simulink library "inp"  (Simulink 8.2)
settings: Solver=ode45  SolverMode=SingleTasking  StartTime=0.0  StopTime=10.0  RelTol=1e-3
undefined symbols (not defined in the file or in its folder): C_TICK_TIME_S, ENUM_FAULTSTATE_FAIL, ENUM_FAULTSTATE_INDETERMINATE, ENUM_FAULTSTATE_PASS, ENUM_KEYSWITCH_ON, FALSE, KINP_ABS1CANCommuOk_flg_ovrdflg, KINP_ABS1CANCommuOk_flg_ovrdval, KINP_ABS1CANPeriodTime_s, KINP_ABS1CANRolCntOk_flg_ovrdflg, KINP_ABS1CANRolCntOk_flg_ovrdval, KINP_ABS2CANChkSumOk_flg_ovrdflg, KINP_ABS2CANChkSumOk_flg_ovrdval, KINP_ABS2CANCommuOk_flg_ovrdflg, KINP_ABS2CANCommuOk_flg_ovrdval, KINP_ABS2CANPeriodTime_s, KINP_ABS2CANRolCntOk_flg_ovrdflg, KINP_ABS2CANRolCntOk_flg_ovrdval, KINP_ABS3CANCommuOk_flg_ovrdflg, KINP_ABS3CANCommuOk_flg_ovrdval, KINP_ABS4CANCommuOk_flg_ovrdflg, KINP_ABS4CANCommuOk_flg_ovrdval, KINP_ABSActive_flg_defval, KINP_ABSActive_flg_invalid, KINP_ABSActive_flg_ovrdflg, KINP_ABSActive_flg_ovrdval, KINP_ABSActive_flg_setvalid, KINP_ABSActive_flg_srvcflg, KINP_ABSActive_flg_srvcval, KINP_ABSCANAlive_flg_ovrdflg, KINP_ABSCANAlive_flg_ovrdval, KINP_ABSCANCommuOk_flg_ovrdflg, KINP_ABSCANCommuOk_flg_ovrdval, KINP_ABSCANValidOk_flg_ovrdflg, KINP_ABSCANValidOk_flg_ovrdval, KINP_ABSFault_flg_defval, KINP_ABSFault_flg_invalid, KINP_ABSFault_flg_ovrdflg, KINP_ABSFault_flg_ovrdval, KINP_ABSFault_flg_setvalid, KINP_ABSFault_flg_srvcflg, KINP_ABSFault_flg_srvcval, KINP_AC1CANCommuOk_flg_ovrdflg, KINP_AC1CANCommuOk_flg_ovrdval, KINP_ACCANAlive_flg_ovrdflg, KINP_ACCANAlive_flg_ovrdval, KINP_ACCANCommuOk_flg_ovrdflg, KINP_ACCANCommuOk_flg_ovrdval, KINP_ACCANValidOk_flg_ovrdflg, KINP_ACCANValidOk_flg_ovrdval, KINP_ACCCancelH_flg_defval, KINP_ACCCancelH_flg_invalid, KINP_ACCCancelH_flg_ovrdflg, KINP_ACCCancelH_flg_ovrdval, KINP_ACCCancelH_flg_setvalid, KINP_ACCCancelH_flg_srvcflg, KINP_ACCCancelH_flg_srvcval, KINP_AccelPedalPosn_pct_defval, KINP_AccelPedalPosn_pct_gain, KINP_AccelPedalPosn_pct_invalid ... (+1866)
libraries: inp x192, hcu_lib x34, simulink x33
(part 19 of 21)

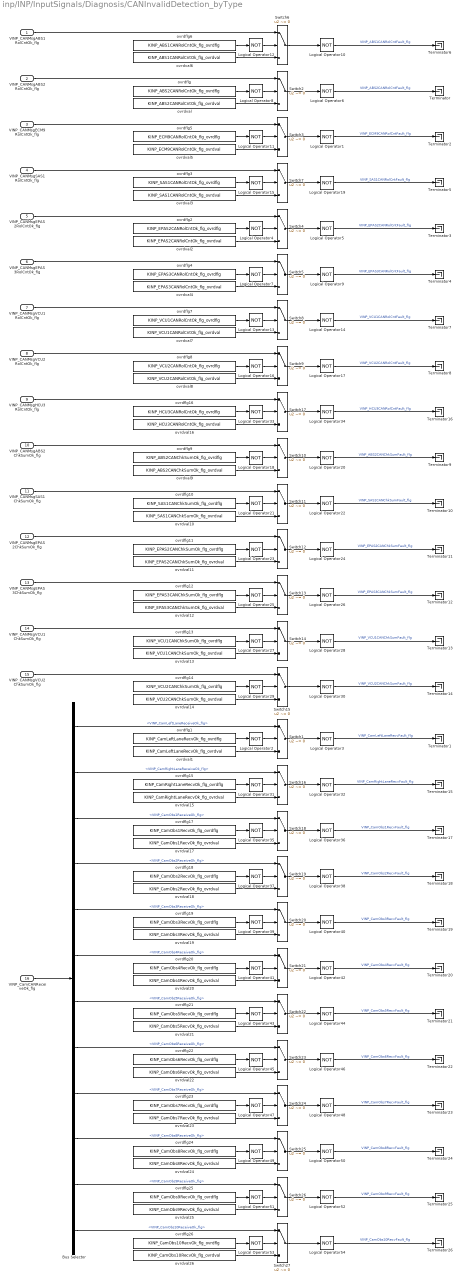
== system inp/INP/InputSignals/Diagnosis/CANInvalidDetection_byType ==
  VINP_CANMsgABS1RolCntOk_flg : Inport
  VINP_CANMsgABS2RolCntOk_flg : Inport  Port=2
  VINP_CANMsgECM9RolCntOk_flg : Inport  Port=3
  VINP_CANMsgSAS1RolCntOk_flg : Inport  Port=4
  VINP_CANMsgEPAS2RolCntOk_flg : Inport  Port=5
  VINP_CANMsgEPAS3RolCntOk_flg : Inport  Port=6
  VINP_CANMsgVCU1RolCntOk_flg : Inport  Port=7
  VINP_CANMsgVCU2RolCntOk_flg : Inport  Port=8
  VINP_CANMsgHCU3RolCntOk_flg : Inport  Port=9
  VINP_CANMsgABS2ChkSumOk_flg : Inport  Port=10
  VINP_CANMsgSAS1ChkSumOk_flg : Inport  Port=11
  VINP_CANMsgEPAS2ChkSumOk_flg : Inport  Port=12
  VINP_CANMsgEPAS3ChkSumOk_flg : Inport  Port=13
  VINP_CANMsgVCU1ChkSumOk_flg : Inport  Port=14
  VINP_CANMsgVCU2ChkSumOk_flg : Inport  Port=15
  VINP_CamCANReceiveOk_flg : Inport  Port=16
  Bus Selector : BusSelector  OutputSignals=VINP_CamLeftLaneReceiveOk_flg,VINP_CamRightLaneReceiveOk_flg,VINP_CamObs1ReceiveOk_flg,VINP_CamObs2ReceiveOk_flg,VINP_CamObs3ReceiveOk_flg,VINP_CamObs4ReceiveOk_flg,VINP_CamObs5ReceiveOk_flg,VINP_CamObs6ReceiveOk_flg,VINP_CamObs7ReceiveOk_flg,VINP_CamObs8ReceiveOk_flg,VINP_CamObs9ReceiveOk_flg,VINP_CamObs10ReceiveOk_flg
  Logical Operator{1..54} (54 alike) : Logic  Operator=NOT  AllPortsSameDT=off  OutDataTypeStr=boolean
  Switch{1..27} (27 alike) : Switch  Criteria=u2 ~= 0
  Terminator{,1..26} (27 alike) : Terminator
  ovrdflg : Constant  Value=KINP_ABS2CANRolCntOk_flg_ovrdflg
  ovrdflg1 : Constant  Value=KINP_CamLeftLaneRecvOk_flg_ovrdflg
  ovrdflg10 : Constant  Value=KINP_SAS1CANChkSumOk_flg_ovrdflg
  ovrdflg11 : Constant  Value=KINP_EPAS2CANChkSumOk_flg_ovrdflg
  ovrdflg12 : Constant  Value=KINP_EPAS3CANChkSumOk_flg_ovrdflg
  ovrdflg13 : Constant  Value=KINP_VCU1CANChkSumOk_flg_ovrdflg
  ovrdflg14 : Constant  Value=KINP_VCU2CANChkSumOk_flg_ovrdflg
  ovrdflg15 : Constant  Value=KINP_CamRightLaneRecvOk_flg_ovrdflg
  ovrdflg16 : Constant  Value=KINP_HCU3CANRolCntOk_flg_ovrdflg
  ovrdflg17 : Constant  Value=KINP_CamObs1RecvOk_flg_ovrdflg
  ovrdflg18 : Constant  Value=KINP_CamObs2RecvOk_flg_ovrdflg
  ovrdflg19 : Constant  Value=KINP_CamObs3RecvOk_flg_ovrdflg
  ovrdflg2 : Constant  Value=KINP_EPAS2CANRolCntOk_flg_ovrdflg
  ovrdflg20 : Constant  Value=KINP_CamObs4RecvOk_flg_ovrdflg
  ovrdflg21 : Constant  Value=KINP_CamObs5RecvOk_flg_ovrdflg
  ovrdflg22 : Constant  Value=KINP_CamObs6RecvOk_flg_ovrdflg
  ovrdflg23 : Constant  Value=KINP_CamObs7RecvOk_flg_ovrdflg
  ovrdflg24 : Constant  Value=KINP_CamObs8RecvOk_flg_ovrdflg
  ovrdflg25 : Constant  Value=KINP_CamObs9RecvOk_flg_ovrdflg
  ovrdflg26 : Constant  Value=KINP_CamObs10RecvOk_flg_ovrdflg
  ovrdflg3 : Constant  Value=KINP_SAS1CANRolCntOk_flg_ovrdflg
  ovrdflg4 : Constant  Value=KINP_EPAS3CANRolCntOk_flg_ovrdflg
  ovrdflg5 : Constant  Value=KINP_ECM9CANRolCntOk_flg_ovrdflg
  ovrdflg6 : Constant  Value=KINP_ABS1CANRolCntOk_flg_ovrdflg
  ovrdflg7 : Constant  Value=KINP_VCU1CANRolCntOk_flg_ovrdflg
  ovrdflg8 : Constant  Value=KINP_VCU2CANRolCntOk_flg_ovrdflg
  ovrdflg9 : Constant  Value=KINP_ABS2CANChkSumOk_flg_ovrdflg
  ovrdval : Constant  Value=KINP_ABS2CANRolCntOk_flg_ovrdval
  ovrdval1 : Constant  Value=KINP_CamLeftLaneRecvOk_flg_ovrdval
  ovrdval10 : Constant  Value=KINP_SAS1CANChkSumOk_flg_ovrdval
  ovrdval11 : Constant  Value=KINP_EPAS2CANChkSumOk_flg_ovrdval
  ovrdval12 : Constant  Value=KINP_EPAS3CANChkSumOk_flg_ovrdval
  ovrdval13 : Constant  Value=KINP_VCU1CANChkSumOk_flg_ovrdval
  ovrdval14 : Constant  Value=KINP_VCU2CANChkSumOk_flg_ovrdval
  ovrdval15 : Constant  Value=KINP_CamRightLaneRecvOk_flg_ovrdval
  ovrdval16 : Constant  Value=KINP_HCU3CANRolCntOk_flg_ovrdval
  ovrdval17 : Constant  Value=KINP_CamObs1RecvOk_flg_ovrdval
  ovrdval18 : Constant  Value=KINP_CamObs2RecvOk_flg_ovrdval
  ovrdval19 : Constant  Value=KINP_CamObs3RecvOk_flg_ovrdval
  ovrdval2 : Constant  Value=KINP_EPAS2CANRolCntOk_flg_ovrdval
  ovrdval20 : Constant  Value=KINP_CamObs4RecvOk_flg_ovrdval
  ovrdval21 : Constant  Value=KINP_CamObs5RecvOk_flg_ovrdval
  ovrdval22 : Constant  Value=KINP_CamObs6RecvOk_flg_ovrdval
  ovrdval23 : Constant  Value=KINP_CamObs7RecvOk_flg_ovrdval
  ovrdval24 : Constant  Value=KINP_CamObs8RecvOk_flg_ovrdval
  ovrdval25 : Constant  Value=KINP_CamObs9RecvOk_flg_ovrdval
  ovrdval26 : Constant  Value=KINP_CamObs10RecvOk_flg_ovrdval
  ovrdval3 : Constant  Value=KINP_SAS1CANRolCntOk_flg_ovrdval
  ovrdval4 : Constant  Value=KINP_EPAS3CANRolCntOk_flg_ovrdval
  ovrdval5 : Constant  Value=KINP_ECM9CANRolCntOk_flg_ovrdval
  ovrdval6 : Constant  Value=KINP_ABS1CANRolCntOk_flg_ovrdval
  ovrdval7 : Constant  Value=KINP_VCU1CANRolCntOk_flg_ovrdval
  ovrdval8 : Constant  Value=KINP_VCU2CANRolCntOk_flg_ovrdval
  ovrdval9 : Constant  Value=KINP_ABS2CANChkSumOk_flg_ovrdval
  wires:
  VINP_CANMsgSAS1RolCntOk_flg/1 -> Switch7/1
  VINP_CANMsgECM9RolCntOk_flg/1 -> Switch3/1
  VINP_CANMsgABS2RolCntOk_flg/1 -> Switch2/1
  Switch7/1 -> Logical Operator19/1
  ovrdflg3/1 -> Logical Operator15/1
  ovrdval3/1 -> Switch7/3
  Logical Operator15/1 -> Switch7/2
  Switch2/1 -> Logical Operator6/1
  Switch3/1 -> Logical Operator1/1
  ovrdflg/1 -> Logical Operator8/1
  ovrdval/1 -> Switch2/3
  ovrdflg5/1 -> Logical Operator11/1
  ovrdval5/1 -> Switch3/3
  Logical Operator8/1 -> Switch2/2
  Logical Operator11/1 -> Switch3/2
  Logical Operator6/1 -> Terminator/1  "VINP_ABS2CANRolCntFault_flg"
  Logical Operator1/1 -> Terminator2/1  "VINP_ECM9CANRolCntFault_flg"
  Logical Operator19/1 -> Terminator5/1  "VINP_SAS1CANRolCntFault_flg"
  VINP_CANMsgEPAS2RolCntOk_flg/1 -> Switch4/1
  Switch4/1 -> Logical Operator5/1
  ovrdflg2/1 -> Logical Operator4/1
  ovrdval2/1 -> Switch4/3
  Logical Operator4/1 -> Switch4/2
  Logical Operator5/1 -> Terminator3/1  "VINP_EPAS2CANRolCntFault_flg"
  VINP_CANMsgEPAS3RolCntOk_flg/1 -> Switch5/1
  Switch5/1 -> Logical Operator9/1
  ovrdflg4/1 -> Logical Operator7/1
  ovrdval4/1 -> Switch5/3
  Logical Operator7/1 -> Switch5/2
  Logical Operator9/1 -> Terminator4/1  "VINP_EPAS3CANRolCntFault_flg"
  VINP_CANMsgABS1RolCntOk_flg/1 -> Switch6/1
  Switch6/1 -> Logical Operator10/1
  ovrdflg6/1 -> Logical Operator12/1
  ovrdval6/1 -> Switch6/3
  Logical Operator12/1 -> Switch6/2
  Logical Operator10/1 -> Terminator6/1  "VINP_ABS1CANRolCntFault_flg"
  VINP_CANMsgVCU1RolCntOk_flg/1 -> Switch8/1
  Switch8/1 -> Logical Operator14/1
  ovrdflg7/1 -> Logical Operator13/1
  ovrdval7/1 -> Switch8/3
  Logical Operator13/1 -> Switch8/2
  Logical Operator14/1 -> Terminator7/1  "VINP_VCU1CANRolCntFault_flg"
  VINP_CANMsgVCU2RolCntOk_flg/1 -> Switch9/1
  Switch9/1 -> Logical Operator17/1
  ovrdflg8/1 -> Logical Operator16/1
  ovrdval8/1 -> Switch9/3
  Logical Operator16/1 -> Switch9/2
  Logical Operator17/1 -> Terminator8/1  "VINP_VCU2CANRolCntFault_flg"
  VINP_CANMsgABS2ChkSumOk_flg/1 -> Switch10/1
  Switch10/1 -> Logical Operator20/1
  ovrdflg9/1 -> Logical Operator18/1
  ovrdval9/1 -> Switch10/3
  Logical Operator18/1 -> Switch10/2
  Logical Operator20/1 -> Terminator9/1  "VINP_ABS2CANChkSumFault_flg"
  VINP_CANMsgSAS1ChkSumOk_flg/1 -> Switch11/1
  Switch11/1 -> Logical Operator22/1
  ovrdflg10/1 -> Logical Operator21/1
  ovrdval10/1 -> Switch11/3
  Logical Operator21/1 -> Switch11/2
  Logical Operator22/1 -> Terminator10/1  "VINP_SAS1CANChkSumFault_flg"
  VINP_CANMsgEPAS2ChkSumOk_flg/1 -> Switch12/1
  Switch12/1 -> Logical Operator24/1
  ovrdflg11/1 -> Logical Operator23/1
  ovrdval11/1 -> Switch12/3
  Logical Operator23/1 -> Switch12/2
  Logical Operator24/1 -> Terminator11/1  "VINP_EPAS2CANChkSumFault_flg"
  VINP_CANMsgEPAS3ChkSumOk_flg/1 -> Switch13/1
  Switch13/1 -> Logical Operator26/1
  ovrdflg12/1 -> Logical Operator25/1
  ovrdval12/1 -> Switch13/3
  Logical Operator25/1 -> Switch13/2
  Logical Operator26/1 -> Terminator12/1  "VINP_EPAS3CANChkSumFault_flg"
  VINP_CANMsgVCU1ChkSumOk_flg/1 -> Switch14/1
  Switch14/1 -> Logical Operator28/1
  ovrdflg13/1 -> Logical Operator27/1
  ovrdval13/1 -> Switch14/3
  Logical Operator27/1 -> Switch14/2
  Logical Operator28/1 -> Terminator13/1  "VINP_VCU1CANChkSumFault_flg"
  VINP_CANMsgVCU2ChkSumOk_flg/1 -> Switch15/1
  Switch15/1 -> Logical Operator30/1
  ovrdflg14/1 -> Logical Operator29/1
  ovrdval14/1 -> Switch15/3
  Logical Operator29/1 -> Switch15/2
  Logical Operator30/1 -> Terminator14/1  "VINP_VCU2CANChkSumFault_flg"
  VINP_CANMsgHCU3RolCntOk_flg/1 -> Switch17/1
  Switch17/1 -> Logical Operator34/1
  ovrdflg16/1 -> Logical Operator33/1
  ovrdval16/1 -> Switch17/3
  Logical Operator33/1 -> Switch17/2
  Logical Operator34/1 -> Terminator16/1  "VINP_HCU3CANRolCntFault_flg"
  Switch1/1 -> Logical Operator3/1
  ovrdflg1/1 -> Logical Operator2/1
  ovrdval1/1 -> Switch1/3
  Logical Operator2/1 -> Switch1/2
  Logical Operator3/1 -> Terminator1/1  "VINP_CamLeftLaneRecvFault_flg"
  Bus Selector/1 -> Switch1/1  "<VINP_CamLeftLaneReceiveOk_flg>"
  Switch16/1 -> Logical Operator32/1
  ovrdflg15/1 -> Logical Operator31/1
  ovrdval15/1 -> Switch16/3
  Logical Operator31/1 -> Switch16/2
  Logical Operator32/1 -> Terminator15/1  "VINP_CamRightLaneRecvFault_flg"
  Bus Selector/2 -> Switch16/1  "<VINP_CamRightLaneReceiveOk_flg>"
  VINP_CamCANReceiveOk_flg/1 -> Bus Selector/1
  Switch18/1 -> Logical Operator36/1
  ovrdflg17/1 -> Logical Operator35/1
  ovrdval17/1 -> Switch18/3
  Logical Operator35/1 -> Switch18/2
  Logical Operator36/1 -> Terminator17/1  "VINP_CamObs1RecvFault_flg"
  Bus Selector/3 -> Switch18/1  "<VINP_CamObs1ReceiveOk_flg>"
  Switch19/1 -> Logical Operator38/1
  ovrdflg18/1 -> Logical Operator37/1
  ovrdval18/1 -> Switch19/3
  Logical Operator37/1 -> Switch19/2
  Logical Operator38/1 -> Terminator18/1  "VINP_CamObs2RecvFault_flg"
  Bus Selector/4 -> Switch19/1  "<VINP_CamObs2ReceiveOk_flg>"
  Switch20/1 -> Logical Operator40/1
  ovrdflg19/1 -> Logical Operator39/1
  ovrdval19/1 -> Switch20/3
  Logical Operator39/1 -> Switch20/2
  Logical Operator40/1 -> Terminator19/1  "VINP_CamObs3RecvFault_flg"
  Bus Selector/5 -> Switch20/1  "<VINP_CamObs3ReceiveOk_flg>"
  Switch21/1 -> Logical Operator42/1
  ovrdflg20/1 -> Logical Operator41/1
  ovrdval20/1 -> Switch21/3
  Logical Operator41/1 -> Switch21/2
  Logical Operator42/1 -> Terminator20/1  "VINP_CamObs4RecvFault_flg"
  Bus Selector/6 -> Switch21/1  "<VINP_CamObs4ReceiveOk_flg>"
  Switch22/1 -> Logical Operator44/1
  ovrdflg21/1 -> Logical Operator43/1
  ovrdval21/1 -> Switch22/3
  Logical Operator43/1 -> Switch22/2
  Logical Operator44/1 -> Terminator21/1  "VINP_CamObs5RecvFault_flg"
  Bus Selector/7 -> Switch22/1  "<VINP_CamObs5ReceiveOk_flg>"
  Switch23/1 -> Logical Operator46/1
  ovrdflg22/1 -> Logical Operator45/1
  ovrdval22/1 -> Switch23/3
  Logical Operator45/1 -> Switch23/2
  Logical Operator46/1 -> Terminator22/1  "VINP_CamObs6RecvFault_flg"
  Bus Selector/8 -> Switch23/1  "<VINP_CamObs6ReceiveOk_flg>"
  Switch24/1 -> Logical Operator48/1
  ovrdflg23/1 -> Logical Operator47/1
  ovrdval23/1 -> Switch24/3
  Logical Operator47/1 -> Switch24/2
  Logical Operator48/1 -> Terminator23/1  "VINP_CamObs7RecvFault_flg"
  Bus Selector/9 -> Switch24/1  "<VINP_CamObs7ReceiveOk_flg>"
  Switch25/1 -> Logical Operator50/1
  ovrdflg24/1 -> Logical Operator49/1
  ovrdval24/1 -> Switch25/3
  Logical Operator49/1 -> Switch25/2
  Logical Operator50/1 -> Terminator24/1  "VINP_CamObs8RecvFault_flg"
  Bus Selector/10 -> Switch25/1  "<VINP_CamObs8ReceiveOk_flg>"
  Switch26/1 -> Logical Operator52/1
  ovrdflg25/1 -> Logical Operator51/1
  ovrdval25/1 -> Switch26/3
  Logical Operator51/1 -> Switch26/2
  Logical Operator52/1 -> Terminator25/1  "VINP_CamObs9RecvFault_flg"
  Bus Selector/11 -> Switch26/1  "<VINP_CamObs9ReceiveOk_flg>"
  Switch27/1 -> Logical Operator54/1
  ovrdflg26/1 -> Logical Operator53/1
  ovrdval26/1 -> Switch27/3
  Logical Operator53/1 -> Switch27/2
  Logical Operator54/1 -> Terminator26/1  "VINP_CamObs10RecvFault_flg"
  Bus Selector/12 -> Switch27/1  "<VINP_CamObs10ReceiveOk_flg>"

== system inp/INP/InputSignals/Diagnosis/ECM_CANSig_Detection ==
  VINP_AccelPedalPosnSigOk_flg_InHAL : Inport
  VINP_EffAccelPedalPosnSigOk_flg_InHAL : Inport  Port=2
  VINP_EngSpdSigOk_flg_InHAL : Inport  Port=3
  VINP_EngTqFrictionSigOk_flg_InHAL : Inport  Port=4
  VINP_EngTqIndicatedSigOk_flg_InHAL : Inport  Port=5
  VINP_EngTqIndicatedCORSigOk_flg_InHAL : Inport  Port=6
  VINP_EngTqMaxSigOk_flg_InHAL : Inport  Port=7
  VINP_EngTqMinSigOk_flg_InHAL : Inport  Port=8
  Logical Operator{1..5,7,9..14,16..18,20} (16 alike) : Logic  Operator=NOT  AllPortsSameDT=off  OutDataTypeStr=boolean
  Switch{1,3..6,8..10} (8 alike) : Switch  Criteria=u2 ~= 0
  Terminator{1..4,6..9} (8 alike) : Terminator
  ovrdflg1 : Constant  Value=KINP_CANEffAPPSigOk_flg_ovrdflg
  ovrdflg2 : Constant  Value=KINP_CANEngSpdSigOk_flg_ovrdflg
  ovrdflg4 : Constant  Value=KINP_CANEngTqFricSigOk_flg_ovrdflg
  ovrdflg5 : Constant  Value=KINP_CANAPPSigOk_flg_ovrdflg
  ovrdflg6 : Constant  Value=KINP_CANEngTqMaxSigOk_flg_ovrdflg
  ovrdflg7 : Constant  Value=KINP_CANEngTqMinSigOk_flg_ovrdflg
  ovrdflg8 : Constant  Value=KINP_CANEngTqIndSigOk_flg_ovrdflg
  ovrdflg9 : Constant  Value=KINP_CANEngTqIndCORSigOk_flg_ovrdflg
  ovrdval1 : Constant  Value=KINP_CANEffAPPSigOk_flg_ovrdval
  ovrdval2 : Constant  Value=KINP_CANEngSpdSigOk_flg_ovrdval
  ovrdval4 : Constant  Value=KINP_CANEngTqFricSigOk_flg_ovrdval
  ovrdval5 : Constant  Value=KINP_CANAPPSigOk_flg_ovrdval
  ovrdval6 : Constant  Value=KINP_CANEngTqMaxSigOk_flg_ovrdval
  ovrdval7 : Constant  Value=KINP_CANEngTqMinSigOk_flg_ovrdval
  ovrdval8 : Constant  Value=KINP_CANEngTqIndSigOk_flg_ovrdval
  ovrdval9 : Constant  Value=KINP_CANEngTqIndCORSigOk_flg_ovrdval
  wires:
  Logical Operator1/1 -> Terminator2/1  "VINP_CANAPPSigFault_flg"
  Logical Operator11/1 -> Switch3/2
  ovrdval5/1 -> Switch3/3
  ovrdflg5/1 -> Logical Operator11/1
  Switch3/1 -> Logical Operator1/1
  VINP_AccelPedalPosnSigOk_flg_InHAL/1 -> Switch3/1
  Logical Operator2/1 -> Terminator1/1  "VINP_CANEffAPPSigFault_flg"
  Logical Operator3/1 -> Switch1/2
  ovrdval1/1 -> Switch1/3
  ovrdflg1/1 -> Logical Operator3/1
  Switch1/1 -> Logical Operator2/1
  VINP_EffAccelPedalPosnSigOk_flg_InHAL/1 -> Switch1/1
  Logical Operator4/1 -> Terminator3/1  "VINP_CANEngSpdSigFault_flg"
  Logical Operator5/1 -> Switch4/2
  ovrdval2/1 -> Switch4/3
  ovrdflg2/1 -> Logical Operator5/1
  Switch4/1 -> Logical Operator4/1
  VINP_EngSpdSigOk_flg_InHAL/1 -> Switch4/1
  Logical Operator7/1 -> Terminator4/1  "VINP_CANEngTqFricSigFault_flg"
  Logical Operator9/1 -> Switch5/2
  ovrdval4/1 -> Switch5/3
  ovrdflg4/1 -> Logical Operator9/1
  Switch5/1 -> Logical Operator7/1
  VINP_EngTqFrictionSigOk_flg_InHAL/1 -> Switch5/1
  Logical Operator10/1 -> Terminator6/1  "VINP_CANEngTqMaxSigFault_flg"
  Logical Operator12/1 -> Switch6/2
  ovrdval6/1 -> Switch6/3
  ovrdflg6/1 -> Logical Operator12/1
  Switch6/1 -> Logical Operator10/1
  VINP_EngTqMaxSigOk_flg_InHAL/1 -> Switch6/1
  Logical Operator13/1 -> Terminator7/1  "VINP_CANEngTqMinSigFault_flg"
  Logical Operator14/1 -> Switch8/2
  ovrdval7/1 -> Switch8/3
  ovrdflg7/1 -> Logical Operator14/1
  Switch8/1 -> Logical Operator13/1
  VINP_EngTqMinSigOk_flg_InHAL/1 -> Switch8/1
  Logical Operator16/1 -> Terminator8/1  "VINP_CANEngTqIndSigFault_flg"
  Logical Operator17/1 -> Switch9/2
  ovrdval8/1 -> Switch9/3
  ovrdflg8/1 -> Logical Operator17/1
  Switch9/1 -> Logical Operator16/1
  VINP_EngTqIndicatedSigOk_flg_InHAL/1 -> Switch9/1
  Logical Operator18/1 -> Terminator9/1  "VINP_CANEngTqIndCORSigFault_flg"
  Logical Operator20/1 -> Switch10/2
  ovrdval9/1 -> Switch10/3
  ovrdflg9/1 -> Logical Operator20/1
  Switch10/1 -> Logical Operator18/1
  VINP_EngTqIndicatedCORSigOk_flg_InHAL/1 -> Switch10/1

== system inp/INP/InputSignals/Diagnosis/EPB_CANSig_Detection ==
  VINP_ParkBrakeBindStaSigOk_flg_InHAL : Inport
  Logical Operator6 : Logic  Operator=NOT  AllPortsSameDT=off  OutDataTypeStr=boolean
  Logical Operator8 : Logic  Operator=NOT  AllPortsSameDT=off  OutDataTypeStr=boolean
  Switch2 : Switch  Criteria=u2 ~= 0
  Terminator : Terminator
  ovrdflg : Constant  Value=KINP_CANParkBrakBindStaSigOk_flg_ovrdflg
  ovrdval : Constant  Value=KINP_CANParkBrakBindStaSigOk_flg_ovrdval
  wires:
  Logical Operator6/1 -> Terminator/1  "VINP_CANParkBrakBindStaSigFault_flg"
  Logical Operator8/1 -> Switch2/2
  ovrdval/1 -> Switch2/3
  ovrdflg/1 -> Logical Operator8/1
  Switch2/1 -> Logical Operator6/1
  VINP_ParkBrakeBindStaSigOk_flg_InHAL/1 -> Switch2/1

== system inp/INP/InputSignals/Diagnosis/EPS_CANSig_Detection ==
  VINP_SteerAngleSigOk_flg_InHAL : Inport
  VINP_SteerSpeedSigOk_flg_InHAL : Inport  Port=2
  VINP_RelSteerAngleSigOk_flg_InHAL : Inport  Port=3
  VINP_SteerTorqueSigOk_flg_InHAL : Inport  Port=4
  VINP_AckCurStrAngReqSigOk_flg_InHAL : Inport  Port=5
  VINP_AckCurStrTqReqSigOk_flg_InHAL : Inport  Port=6
  VINP_EPSBattVoltSigOk_flg_InHAL : Inport  Port=7
  Logical Operator{1..14} (14 alike) : Logic  Operator=NOT  AllPortsSameDT=off  OutDataTypeStr=boolean
  Switch{1..7} (7 alike) : Switch  Criteria=u2 ~= 0
  Terminator{,1..6} (7 alike) : Terminator
  ovrdflg : Constant  Value=KINP_CANStrAngSigOk_flg_ovrdflg
  ovrdflg1 : Constant  Value=KINP_CANStrTorqSigOk_flg_ovrdflg
  ovrdflg2 : Constant  Value=KINP_CANRelStrAngSigOk_flg_ovrdflg
  ovrdflg3 : Constant  Value=KINP_CANAckCurStrAngReqSigOk_flg_ovrdflg
  ovrdflg4 : Constant  Value=KINP_CANAckCurStrTqReqSigOk_flg_ovrdflg
  ovrdflg5 : Constant  Value=KINP_CANEPSBattVoltSigOk_flg_ovrdflg
  ovrdflg6 : Constant  Value=KINP_CANStrSpdSigOk_flg_ovrdflg
  ovrdval : Constant  Value=KINP_CANStrAngSigOk_flg_ovrdval
  ovrdval1 : Constant  Value=KINP_CANStrTorqSigOk_flg_ovrdval
  ovrdval2 : Constant  Value=KINP_CANRelStrAngSigOk_flg_ovrdval
  ovrdval3 : Constant  Value=KINP_CANAckCurStrAngReqSigOk_flg_ovrdval
  ovrdval4 : Constant  Value=KINP_CANAckCurStrTqReqSigOk_flg_ovrdval
  ovrdval5 : Constant  Value=KINP_CANEPSBattVoltSigOk_flg_ovrdval
  ovrdval6 : Constant  Value=KINP_CANStrSpdSigOk_flg_ovrdval
  wires:
  VINP_SteerAngleSigOk_flg_InHAL/1 -> Switch2/1
  Switch2/1 -> Logical Operator6/1
  ovrdflg/1 -> Logical Operator8/1
  ovrdval/1 -> Switch2/3
  Logical Operator8/1 -> Switch2/2
  Logical Operator6/1 -> Terminator/1  "VINP_CANStrAngSigFault_flg"
  VINP_SteerTorqueSigOk_flg_InHAL/1 -> Switch1/1
  Switch1/1 -> Logical Operator1/1
  ovrdflg1/1 -> Logical Operator2/1
  ovrdval1/1 -> Switch1/3
  Logical Operator2/1 -> Switch1/2
  Logical Operator1/1 -> Terminator1/1  "VINP_CANStrTorqSigFault_flg"
  VINP_RelSteerAngleSigOk_flg_InHAL/1 -> Switch3/1
  Switch3/1 -> Logical Operator3/1
  ovrdflg2/1 -> Logical Operator4/1
  ovrdval2/1 -> Switch3/3
  Logical Operator4/1 -> Switch3/2
  Logical Operator3/1 -> Terminator2/1  "VINP_CANRelStrAngSigFault_flg"
  VINP_AckCurStrAngReqSigOk_flg_InHAL/1 -> Switch4/1
  Switch4/1 -> Logical Operator5/1
  ovrdflg3/1 -> Logical Operator7/1
  ovrdval3/1 -> Switch4/3
  Logical Operator7/1 -> Switch4/2
  Logical Operator5/1 -> Terminator3/1  "VINP_CANAckCurStrAngReqSigFault_flg"
  VINP_AckCurStrTqReqSigOk_flg_InHAL/1 -> Switch5/1
  Switch5/1 -> Logical Operator9/1
  ovrdflg4/1 -> Logical Operator10/1
  ovrdval4/1 -> Switch5/3
  Logical Operator10/1 -> Switch5/2
  Logical Operator9/1 -> Terminator4/1  "VINP_CANAckCurStrTqReqSigFault_flg"
  VINP_EPSBattVoltSigOk_flg_InHAL/1 -> Switch6/1
  Switch6/1 -> Logical Operator12/1
  ovrdflg5/1 -> Logical Operator11/1
  ovrdval5/1 -> Switch6/3
  Logical Operator11/1 -> Switch6/2
  Logical Operator12/1 -> Terminator5/1  "VINP_CANEPSBattVoltSigFault_flg"
  VINP_SteerSpeedSigOk_flg_InHAL/1 -> Switch7/1
  Switch7/1 -> Logical Operator13/1
  ovrdflg6/1 -> Logical Operator14/1
  ovrdval6/1 -> Switch7/3
  Logical Operator14/1 -> Switch7/2
  Logical Operator13/1 -> Terminator6/1  "VINP_CANStrSpdSigFault_flg"

== system inp/INP/InputSignals/Diagnosis/HWIO_Detection ==
  VINP_IgnitionVoltFltRangeHigh_enum_InHAL : Inport
  VINP_IgnitionVoltFltRangeLow_enum_InHAL : Inport  Port=2
  VINP_BatteryVoltFltRangeHigh_enum_InHAL : Inport  Port=3
  VINP_BatteryVoltFltRangeLow_enum_InHAL : Inport  Port=4
  Compare To Constant{,1..3} (4 alike) : simulink/Logic and Bit Operations/Compare To Constant  relop=~=  const=ENUM_FAULTSTATE_FAIL  OutDataTypeStr=uint8  ZeroCross=off
  Logical Operator{1..5,11,15,19} (8 alike) : Logic  Operator=NOT  AllPortsSameDT=off  OutDataTypeStr=boolean
  Switch{1..3,7} (4 alike) : Switch  Criteria=u2 ~= 0
  Terminator{1..3,5} (4 alike) : Terminator
  ovrdflg1 : Constant  Value=KINP_BattVoltRangHighOk_flg_ovrdflg
  ovrdflg2 : Constant  Value=KINP_BattVoltRangLowOk_flg_ovrdflg
  ovrdflg3 : Constant  Value=KINP_IgnVoltRangLowOk_flg_ovrdflg
  ovrdflg5 : Constant  Value=KINP_IgnVoltRangHighOk_flg_ovrdflg
  ovrdval1 : Constant  Value=KINP_BattVoltRangHighOk_flg_ovrdval
  ovrdval2 : Constant  Value=KINP_BattVoltRangLowOk_flg_ovrdval
  ovrdval3 : Constant  Value=KINP_IgnVoltRangLowOk_flg_ovrdval
  ovrdval5 : Constant  Value=KINP_IgnVoltRangHighOk_flg_ovrdval
  wires:
  VINP_IgnitionVoltFltRangeLow_enum_InHAL/1 -> Compare To Constant1/1
  VINP_IgnitionVoltFltRangeHigh_enum_InHAL/1 -> Compare To Constant/1
  Switch7/1 -> Logical Operator19/1
  ovrdflg3/1 -> Logical Operator15/1
  ovrdval3/1 -> Switch7/3
  Logical Operator15/1 -> Switch7/2
  Switch3/1 -> Logical Operator1/1
  ovrdflg5/1 -> Logical Operator11/1
  ovrdval5/1 -> Switch3/3
  Logical Operator11/1 -> Switch3/2
  Logical Operator1/1 -> Terminator2/1  "VINP_IgnVoltRangHighFault_flg"
  Logical Operator19/1 -> Terminator5/1  "VINP_IgnVoltRangLowFault_flg"
  Compare To Constant/1 -> Switch3/1
  Compare To Constant1/1 -> Switch7/1
  VINP_BatteryVoltFltRangeHigh_enum_InHAL/1 -> Compare To Constant2/1
  Switch1/1 -> Logical Operator2/1
  ovrdflg1/1 -> Logical Operator3/1
  ovrdval1/1 -> Switch1/3
  Logical Operator3/1 -> Switch1/2
  Logical Operator2/1 -> Terminator1/1  "VINP_BattVoltRangHighFault_flg"
  Compare To Constant2/1 -> Switch1/1
  VINP_BatteryVoltFltRangeLow_enum_InHAL/1 -> Compare To Constant3/1
  Switch2/1 -> Logical Operator4/1
  ovrdflg2/1 -> Logical Operator5/1
  ovrdval2/1 -> Switch2/3
  Logical Operator5/1 -> Switch2/2
  Logical Operator4/1 -> Terminator3/1  "VINP_BattVoltRangLowFault_flg"
  Compare To Constant3/1 -> Switch2/1

== system inp/INP/InputSignals/Diagnosis/LLSW_Detection ==
  VHAL_ADCFault_enum : Inport
  VHAL_EEPROMFault_enum : Inport  Port=2
  VHAL_RAMFault_enum : Inport  Port=3
  VHAL_ROMFault_enum : Inport  Port=4
  VHAL_VREF1Fault_enum : Inport  Port=5
  VHAL_VREF2Fault_enum : Inport  Port=6
  Compare To Constant{,1..5} (6 alike) : simulink/Logic and Bit Operations/Compare To Constant  relop=~=  const=ENUM_FAULTSTATE_FAIL  OutDataTypeStr=uint8  ZeroCross=off
  Logical Operator{1..9,11,15,19} (12 alike) : Logic  Operator=NOT  AllPortsSameDT=off  OutDataTypeStr=boolean
  Switch{1..5,7} (6 alike) : Switch  Criteria=u2 ~= 0
  Terminator{1..6} (6 alike) : Terminator
  ovrdflg1 : Constant  Value=KINP_ROMOk_flg_ovrdflg
  ovrdflg2 : Constant  Value=KINP_VREF1Ok_flg_ovrdflg
  ovrdflg3 : Constant  Value=KINP_RAMOk_flg_ovrdflg
  ovrdflg4 : Constant  Value=KINP_VREF2Ok_flg_ovrdflg
  ovrdflg5 : Constant  Value=KINP_EEPROMOk_flg_ovrdflg
  ovrdflg6 : Constant  Value=KINP_ADCOk_flg_ovrdflg
  ovrdval1 : Constant  Value=KINP_ROMOk_flg_ovrdval
  ovrdval2 : Constant  Value=KINP_VREF1Ok_flg_ovrdval
  ovrdval3 : Constant  Value=KINP_RAMOk_flg_ovrdval
  ovrdval4 : Constant  Value=KINP_VREF2Ok_flg_ovrdval
  ovrdval5 : Constant  Value=KINP_EEPROMOk_flg_ovrdval
  ovrdval6 : Constant  Value=KINP_ADCOk_flg_ovrdval
  wires:
  Compare To Constant1/1 -> Switch7/1
  Compare To Constant/1 -> Switch3/1
  Logical Operator19/1 -> Terminator5/1  "VINP_RAMFault_flg"
  Logical Operator1/1 -> Terminator2/1  "VINP_EEPROMFault_flg"
  Logical Operator11/1 -> Switch3/2
  ovrdval5/1 -> Switch3/3
  ovrdflg5/1 -> Logical Operator11/1
  Switch3/1 -> Logical Operator1/1
  Logical Operator15/1 -> Switch7/2
  ovrdval3/1 -> Switch7/3
  ovrdflg3/1 -> Logical Operator15/1
  Switch7/1 -> Logical Operator19/1
  VHAL_EEPROMFault_enum/1 -> Compare To Constant/1
  VHAL_RAMFault_enum/1 -> Compare To Constant1/1
  Compare To Constant2/1 -> Switch1/1
  Logical Operator3/1 -> Terminator1/1  "VINP_ROMFault_flg"
  Logical Operator2/1 -> Switch1/2
  ovrdval1/1 -> Switch1/3
  ovrdflg1/1 -> Logical Operator2/1
  Switch1/1 -> Logical Operator3/1
  VHAL_ROMFault_enum/1 -> Compare To Constant2/1
  Compare To Constant3/1 -> Switch2/1
  Logical Operator5/1 -> Terminator3/1  "VINP_VREF1Fault_flg"
  Logical Operator4/1 -> Switch2/2
  ovrdval2/1 -> Switch2/3
  ovrdflg2/1 -> Logical Operator4/1
  Switch2/1 -> Logical Operator5/1
  VHAL_VREF1Fault_enum/1 -> Compare To Constant3/1
  Compare To Constant4/1 -> Switch4/1
  Logical Operator7/1 -> Terminator4/1  "VINP_VREF2Fault_flg"
  Logical Operator6/1 -> Switch4/2
  ovrdval4/1 -> Switch4/3
  ovrdflg4/1 -> Logical Operator6/1
  Switch4/1 -> Logical Operator7/1
  VHAL_VREF2Fault_enum/1 -> Compare To Constant4/1
  Compare To Constant5/1 -> Switch5/1
  Logical Operator8/1 -> Terminator6/1  "VINP_ADCFault_flg"
  Logical Operator9/1 -> Switch5/2
  ovrdval6/1 -> Switch5/3
  ovrdflg6/1 -> Logical Operator9/1
  Switch5/1 -> Logical Operator8/1
  VHAL_ADCFault_enum/1 -> Compare To Constant5/1

== system inp/INP/InputSignals/Diagnosis/METER_CANSig_Detection ==
  VINP_OdoMeterSigOk_flg_InHAL : Inport
  VINP_MeterVehSpdSigOk_flg_InHAL : Inport  Port=2
  Logical Operator{1..2,6,8} (4 alike) : Logic  Operator=NOT  AllPortsSameDT=off  OutDataTypeStr=boolean
  Switch1 : Switch  Criteria=u2 ~= 0
  Switch2 : Switch  Criteria=u2 ~= 0
  Terminator : Terminator
  Terminator1 : Terminator
  ovrdflg : Constant  Value=KINP_CANOdoMeterSigOk_flg_ovrdflg
  ovrdflg1 : Constant  Value=KINP_CANMeterVehSpdSigOk_flg_ovrdflg
  ovrdval : Constant  Value=KINP_CANOdoMeterSigOk_flg_ovrdval
  ovrdval1 : Constant  Value=KINP_CANMeterVehSpdSigOk_flg_ovrdval
  wires:
  Logical Operator6/1 -> Terminator/1  "VINP_CANOdoMeterSigFault_flg"
  Logical Operator8/1 -> Switch2/2
  ovrdval/1 -> Switch2/3
  ovrdflg/1 -> Logical Operator8/1
  Switch2/1 -> Logical Operator6/1
  VINP_OdoMeterSigOk_flg_InHAL/1 -> Switch2/1
  Logical Operator1/1 -> Terminator1/1  "VINP_CANMeterVehSpdSigFault_flg"
  Logical Operator2/1 -> Switch1/2
  ovrdval1/1 -> Switch1/3
  ovrdflg1/1 -> Logical Operator2/1
  Switch1/1 -> Logical Operator1/1
  VINP_MeterVehSpdSigOk_flg_InHAL/1 -> Switch1/1

== system inp/INP/InputSignals/Diagnosis/Proc_CANAlive ==
  VINP_CANMsgABS1CommuOk_flg : Inport
  VINP_CANMsgABS2CommuOk_flg : Inport  Port=2
  VINP_CANMsgABS3CommuOk_flg : Inport  Port=3
  VINP_CANMsgABS4CommuOk_flg : Inport  Port=4
  VINP_CANMsgBCM1CommuOk_flg : Inport  Port=5
  VINP_CANMsgBCM2CommuOk_flg : Inport  Port=6
  VINP_CANMsgECM1CommuOk_flg : Inport  Port=7
  VINP_CANMsgECM2CommuOk_flg : Inport  Port=8
  VINP_CANMsgECM3CommuOk_flg : Inport  Port=9
  VINP_CANMsgECM9CommuOk_flg : Inport  Port=10
  VINP_CANMsgTCM1CommuOk_flg : Inport  Port=11
  VINP_CANMsgTCM2CommuOk_flg : Inport  Port=12
  VINP_CANMsgSAS1CommuOk_flg : Inport  Port=13
  VINP_CANMsgEPAS1CommuOk_flg : Inport  Port=14
  VINP_CANMsgEPAS2CommuOk_flg : Inport  Port=15
  VINP_CANMsgEPAS3CommuOk_flg : Inport  Port=16
  VINP_CANMsgMETER1CommuOk_flg : Inport  Port=17
  VINP_CANMsgMETER3CommuOk_flg : Inport  Port=18
  VINP_CANMsgAC1CommuOk_flg : Inport  Port=19
  VINP_CANMsgEPB1CommuOk_flg : Inport  Port=20
  VINP_CANMsgVCU1CommuOk_flg : Inport  Port=21
  VINP_CANMsgVCU2CommuOk_flg : Inport  Port=22
  VINP_CANMsgHCU3CommuOk_flg : Inport  Port=23
  VINP_CANMsgHCU4CommuOk_flg : Inport  Port=24
  VINP_CANMsgHMI2CommuOk_flg : Inport  Port=25
  VINP_CANMsgHMI1CommuOk_flg : Inport  Port=26
  VINP_CANMsgCSW1CommuOk_flg : Inport  Port=27
  VINP_CANMsgEPMCommuOk_flg : Inport  Port=28
  Bus Selector : BusSelector  OutputSignals=VINP_CANMsgEPM1CommuOk_flg,VINP_CANMsgEPM2CommuOk_flg,VINP_CANMsgEPM3CommuOk_flg,VINP_CANMsgEPM4CommuOk_flg,VINP_CANMsgEPM5CommuOk_flg,VINP_CANMsgEPM6CommuOk_flg,VINP_CANMsgEPM7CommuOk_flg,VINP_CANMsgEPM8CommuOk_flg,VINP_CANMsgEPM9CommuOk_flg,VINP_CANMsgEPM10CommuOk_flg,VINP_CANMsgEPM11CommuOk_flg,VINP_CANMsgEPM12CommuOk_flg,VINP_CANMsgEPM13CommuOk_flg,VINP_CANMsgEPM14CommuOk_flg,VINP_CANMsgEPM15CommuOk_flg,VINP_CANMsgEPM16CommuOk_flg,VINP_CANMsgEPM17CommuOk_flg,VINP_CANMsgEPM18CommuOk_flg,VINP_CANMsgEPM19CommuOk_flg,VINP_CANMsgEPM20CommuOk_flg,VINP_CANMsgEPM21CommuOk_flg,VINP_CANMsgEPM22CommuOk_flg,VINP_CANMsgEPM23CommuOk_flg,VINP_CANMsgEPM24CommuOk_flg,VINP_CANMsgEPM25CommuOk_flg,VINP_CANMsgEPM26CommuOk_flg,VINP_CANMsgEPM27CommuOk_flg,VINP_CANMsgEPM28CommuOk_flg,VINP_CANMsgEPM29CommuOk_flg,VINP_CANMsgEPM30CommuOk_flg,VINP_CANMsgEPM31CommuOk_flg,VINP_CANMsgEPM32CommuOk_flg,VINP_CANMsgEPM33CommuOk_flg,VINP_CANMsgEPM34CommuOk_flg,VINP_CANMsgEPM35CommuOk_flg
  Logical Operator1 : Logic  Operator=OR  Inputs=4  AllPortsSameDT=off  OutDataTypeStr=boolean
  Logical Operator{6,8..13,15,17,19..20,23} (12 alike) : Logic  Operator=NOT  AllPortsSameDT=off  OutDataTypeStr=boolean
  Logical Operator{14,18,22,24} (4 alike) : Logic  Operator=OR  Inputs=1  AllPortsSameDT=off  OutDataTypeStr=boolean
  Logical Operator16 : Logic  Operator=OR  Inputs=5  AllPortsSameDT=off  OutDataTypeStr=boolean
  Logical Operator2 : Logic  Operator=OR  AllPortsSameDT=off  OutDataTypeStr=boolean
  Logical Operator21 : Logic  Operator=OR  Inputs=35  AllPortsSameDT=off  OutDataTypeStr=boolean
  Logical Operator3 : Logic  Operator=OR  AllPortsSameDT=off  OutDataTypeStr=boolean
  Logical Operator4 : Logic  Operator=OR  Inputs=4  AllPortsSameDT=off  OutDataTypeStr=boolean
  Logical Operator5 : Logic  Operator=OR  AllPortsSameDT=off  OutDataTypeStr=boolean
  Logical Operator7 : Logic  Operator=OR  Inputs=4  AllPortsSameDT=off  OutDataTypeStr=boolean
  Switch{1..12} (12 alike) : Switch  Criteria=u2 ~= 0
  ovrdflg : Constant  Value=KINP_ABSCANAlive_flg_ovrdflg
  ovrdflg1 : Constant  Value=KINP_ACCANAlive_flg_ovrdflg
  ovrdflg10 : Constant  Value=KINP_EPMCANAlive_flg_ovrdflg
  ovrdflg11 : Constant  Value=KINP_CSWCANAlive_flg_ovrdflg
  ovrdflg2 : Constant  Value=KINP_BCMCANAlive_flg_ovrdflg
  ovrdflg3 : Constant  Value=KINP_EPSCANAlive_flg_ovrdflg
  ovrdflg4 : Constant  Value=KINP_EPBCANAlive_flg_ovrdflg
  ovrdflg5 : Constant  Value=KINP_ECMCANAlive_flg_ovrdflg
  ovrdflg6 : Constant  Value=KINP_METERCANAlive_flg_ovrdflg
  ovrdflg7 : Constant  Value=KINP_VCUCANAlive_flg_ovrdflg
  ovrdflg8 : Constant  Value=KINP_TCMCANAlive_flg_ovrdflg
  ovrdflg9 : Constant  Value=KINP_HMICANAlive_flg_ovrdflg
  ovrdval : Constant  Value=KINP_ABSCANAlive_flg_ovrdval
  ovrdval1 : Constant  Value=KINP_ACCANAlive_flg_ovrdval
  ovrdval10 : Constant  Value=KINP_EPMCANAlive_flg_ovrdval
  ovrdval11 : Constant  Value=KINP_CSWCANAlive_flg_ovrdval
  ovrdval2 : Constant  Value=KINP_BCMCANAlive_flg_ovrdval
  ovrdval3 : Constant  Value=KINP_EPSCANAlive_flg_ovrdval
  ovrdval4 : Constant  Value=KINP_EPBCANAlive_flg_ovrdval
  ovrdval5 : Constant  Value=KINP_ECMCANAlive_flg_ovrdval
  ovrdval6 : Constant  Value=KINP_METERCANAlive_flg_ovrdval
  ovrdval7 : Constant  Value=KINP_VCUCANAlive_flg_ovrdval
  ovrdval8 : Constant  Value=KINP_TCMCANAlive_flg_ovrdval
  ovrdval9 : Constant  Value=KINP_HMICANAlive_flg_ovrdval
  VINP_ABSCANAlive_flg_InProc : Outport
  VINP_BCMCANAlive_flg_InProc : Outport  Port=2
  VINP_ECMCANAlive_flg_InProc : Outport  Port=3
  VINP_TCMCANAlive_flg_InProc : Outport  Port=4
  VINP_EPSCANAlive_flg_InProc : Outport  Port=5
  VINP_METERCANAlive_flg_InProc : Outport  Port=6
  VINP_ACCANAlive_flg_InProc : Outport  Port=7
  VINP_EPBCANAlive_flg_InProc : Outport  Port=8
  VINP_VCUCANAlive_flg_InProc : Outport  Port=9
  VINP_HMICANAlive_flg_InProc : Outport  Port=10
  VINP_CSWCANAlive_flg_InProc : Outport  Port=11
  VINP_EPMCANAlive_flg_InProc : Outport  Port=12
  wires:
  Logical Operator12/1 -> Switch4/2
  Logical Operator11/1 -> Switch3/2
  Logical Operator10/1 -> Switch1/2
  Logical Operator8/1 -> Switch2/2
  Logical Operator2/1 -> Switch4/1
  Logical Operator1/1 -> Switch3/1
  Logical Operator5/1 -> Switch1/1
  Logical Operator4/1 -> Switch2/1
  ovrdval8/1 -> Switch4/3
  ovrdflg8/1 -> Logical Operator12/1
  ovrdval5/1 -> Switch3/3
  ovrdflg5/1 -> Logical Operator11/1
  ovrdval2/1 -> Switch1/3
  ovrdflg2/1 -> Logical Operator10/1
  ovrdval/1 -> Switch2/3
  ovrdflg/1 -> Logical Operator8/1
  Switch4/1 -> VINP_TCMCANAlive_flg_InProc/1  "VINP_TCMCANAlive_flg_InProc"
  Switch3/1 -> VINP_ECMCANAlive_flg_InProc/1  "VINP_ECMCANAlive_flg_InProc"
  Switch1/1 -> VINP_BCMCANAlive_flg_InProc/1  "VINP_BCMCANAlive_flg_InProc"
  Switch2/1 -> VINP_ABSCANAlive_flg_InProc/1  "VINP_ABSCANAlive_flg_InProc"
  Logical Operator15/1 -> Switch7/2
  Logical Operator7/1 -> Switch7/1
  ovrdval3/1 -> Switch7/3
  ovrdflg3/1 -> Logical Operator15/1
  Switch7/1 -> VINP_EPSCANAlive_flg_InProc/1  "VINP_EPSCANAlive_flg_InProc"
  VINP_CANMsgSAS1CommuOk_flg/1 -> Logical Operator7/1
  Logical Operator6/1 -> Switch8/2
  Logical Operator3/1 -> Switch8/1
  ovrdval6/1 -> Switch8/3
  ovrdflg6/1 -> Logical Operator6/1
  Switch8/1 -> VINP_METERCANAlive_flg_InProc/1  "VINP_METERCANAlive_flg_InProc"
  VINP_CANMsgBCM1CommuOk_flg/1 -> Logical Operator5/1
  VINP_CANMsgBCM2CommuOk_flg/1 -> Logical Operator5/2
  VINP_CANMsgECM1CommuOk_flg/1 -> Logical Operator1/1
  VINP_CANMsgECM2CommuOk_flg/1 -> Logical Operator1/2
  VINP_CANMsgECM3CommuOk_flg/1 -> Logical Operator1/3
  VINP_CANMsgECM9CommuOk_flg/1 -> Logical Operator1/4
  VINP_CANMsgTCM1CommuOk_flg/1 -> Logical Operator2/1
  VINP_CANMsgTCM2CommuOk_flg/1 -> Logical Operator2/2
  VINP_CANMsgMETER1CommuOk_flg/1 -> Logical Operator3/1
  VINP_CANMsgMETER3CommuOk_flg/1 -> Logical Operator3/2
  Logical Operator13/1 -> Switch5/2
  Logical Operator24/1 -> Switch5/1
  ovrdval1/1 -> Switch5/3
  ovrdflg1/1 -> Logical Operator13/1
  Switch5/1 -> VINP_ACCANAlive_flg_InProc/1  "VINP_ACCANAlive_flg_InProc"
  VINP_CANMsgAC1CommuOk_flg/1 -> Logical Operator24/1
  Logical Operator9/1 -> Switch6/2
  Logical Operator14/1 -> Switch6/1
  ovrdval4/1 -> Switch6/3
  ovrdflg4/1 -> Logical Operator9/1
  Switch6/1 -> VINP_EPBCANAlive_flg_InProc/1  "VINP_EPBCANAlive_flg_InProc"
  VINP_CANMsgEPB1CommuOk_flg/1 -> Logical Operator14/1
  Logical Operator17/1 -> Switch9/2
  Logical Operator16/1 -> Switch9/1
  ovrdval7/1 -> Switch9/3
  ovrdflg7/1 -> Logical Operator17/1
  Switch9/1 -> VINP_VCUCANAlive_flg_InProc/1  "VINP_VCUCANAlive_flg_InProc"
  VINP_CANMsgVCU1CommuOk_flg/1 -> Logical Operator16/1
  VINP_CANMsgVCU2CommuOk_flg/1 -> Logical Operator16/2
  VINP_CANMsgHCU3CommuOk_flg/1 -> Logical Operator16/3
  VINP_CANMsgABS1CommuOk_flg/1 -> Logical Operator4/1
  VINP_CANMsgABS2CommuOk_flg/1 -> Logical Operator4/2
  VINP_CANMsgABS3CommuOk_flg/1 -> Logical Operator4/3
  VINP_CANMsgABS4CommuOk_flg/1 -> Logical Operator4/4
  VINP_CANMsgHCU4CommuOk_flg/1 -> Logical Operator16/4
  VINP_CANMsgHMI2CommuOk_flg/1 -> Logical Operator16/5
  Logical Operator19/1 -> Switch10/2
  Logical Operator18/1 -> Switch10/1
  ovrdval9/1 -> Switch10/3
  ovrdflg9/1 -> Logical Operator19/1
  Switch10/1 -> VINP_HMICANAlive_flg_InProc/1  "VINP_HMICANAlive_flg_InProc"
  VINP_CANMsgHMI1CommuOk_flg/1 -> Logical Operator18/1
  Logical Operator20/1 -> Switch11/2
  Logical Operator21/1 -> Switch11/1
  ovrdval10/1 -> Switch11/3
  ovrdflg10/1 -> Logical Operator20/1
  Switch11/1 -> VINP_EPMCANAlive_flg_InProc/1  "VINP_EPMCANAlive_flg_InProc"
  VINP_CANMsgEPAS1CommuOk_flg/1 -> Logical Operator7/2
  VINP_CANMsgEPAS2CommuOk_flg/1 -> Logical Operator7/3
  VINP_CANMsgEPAS3CommuOk_flg/1 -> Logical Operator7/4
  Logical Operator23/1 -> Switch12/2
  Logical Operator22/1 -> Switch12/1
  ovrdval11/1 -> Switch12/3
  ovrdflg11/1 -> Logical Operator23/1
  Switch12/1 -> VINP_CSWCANAlive_flg_InProc/1  "VINP_CSWCANAlive_flg_InProc"
  VINP_CANMsgCSW1CommuOk_flg/1 -> Logical Operator22/1
  VINP_CANMsgEPMCommuOk_flg/1 -> Bus Selector/1
  Bus Selector/1 -> Logical Operator21/1  "<VINP_CANMsgEPM1CommuOk_flg>"
  Bus Selector/23 -> Logical Operator21/23  "<VINP_CANMsgEPM23CommuOk_flg>"
  Bus Selector/10 -> Logical Operator21/10  "<VINP_CANMsgEPM10CommuOk_flg>"
  Bus Selector/5 -> Logical Operator21/5  "<VINP_CANMsgEPM5CommuOk_flg>"
  Bus Selector/15 -> Logical Operator21/15  "<VINP_CANMsgEPM15CommuOk_flg>"
  Bus Selector/19 -> Logical Operator21/19  "<VINP_CANMsgEPM19CommuOk_flg>"
  Bus Selector/22 -> Logical Operator21/22  "<VINP_CANMsgEPM22CommuOk_flg>"
  Bus Selector/17 -> Logical Operator21/17  "<VINP_CANMsgEPM17CommuOk_flg>"
  Bus Selector/21 -> Logical Operator21/21  "<VINP_CANMsgEPM21CommuOk_flg>"
  Bus Selector/32 -> Logical Operator21/32  "<VINP_CANMsgEPM32CommuOk_flg>"
  Bus Selector/7 -> Logical Operator21/7  "<VINP_CANMsgEPM7CommuOk_flg>"
  Bus Selector/11 -> Logical Operator21/11  "<VINP_CANMsgEPM11CommuOk_flg>"
  Bus Selector/6 -> Logical Operator21/6  "<VINP_CANMsgEPM6CommuOk_flg>"
  Bus Selector/18 -> Logical Operator21/18  "<VINP_CANMsgEPM18CommuOk_flg>"
  Bus Selector/16 -> Logical Operator21/16  "<VINP_CANMsgEPM16CommuOk_flg>"
  Bus Selector/24 -> Logical Operator21/24  "<VINP_CANMsgEPM24CommuOk_flg>"
  Bus Selector/12 -> Logical Operator21/12  "<VINP_CANMsgEPM12CommuOk_flg>"
  Bus Selector/4 -> Logical Operator21/4  "<VINP_CANMsgEPM4CommuOk_flg>"
  Bus Selector/34 -> Logical Operator21/34  "<VINP_CANMsgEPM34CommuOk_flg>"
  Bus Selector/2 -> Logical Operator21/2  "<VINP_CANMsgEPM2CommuOk_flg>"
  Bus Selector/9 -> Logical Operator21/9  "<VINP_CANMsgEPM9CommuOk_flg>"
  Bus Selector/33 -> Logical Operator21/33  "<VINP_CANMsgEPM33CommuOk_flg>"
  Bus Selector/35 -> Logical Operator21/35  "<VINP_CANMsgEPM35CommuOk_flg>"
  Bus Selector/28 -> Logical Operator21/28  "<VINP_CANMsgEPM28CommuOk_flg>"
  Bus Selector/8 -> Logical Operator21/8  "<VINP_CANMsgEPM8CommuOk_flg>"
  Bus Selector/25 -> Logical Operator21/25  "<VINP_CANMsgEPM25CommuOk_flg>"
  Bus Selector/26 -> Logical Operator21/26  "<VINP_CANMsgEPM26CommuOk_flg>"
  Bus Selector/31 -> Logical Operator21/31  "<VINP_CANMsgEPM31CommuOk_flg>"
  Bus Selector/13 -> Logical Operator21/13  "<VINP_CANMsgEPM13CommuOk_flg>"
  Bus Selector/14 -> Logical Operator21/14  "<VINP_CANMsgEPM14CommuOk_flg>"
  Bus Selector/3 -> Logical Operator21/3  "<VINP_CANMsgEPM3CommuOk_flg>"
  Bus Selector/29 -> Logical Operator21/29  "<VINP_CANMsgEPM29CommuOk_flg>"
  Bus Selector/27 -> Logical Operator21/27  "<VINP_CANMsgEPM27CommuOk_flg>"
  Bus Selector/20 -> Logical Operator21/20  "<VINP_CANMsgEPM20CommuOk_flg>"
  Bus Selector/30 -> Logical Operator21/30  "<VINP_CANMsgEPM30CommuOk_flg>"

== system inp/INP/InputSignals/Diagnosis/TCM_CANSig_Detection ==
  VINP_ShifterPosnSigOk_flg_InHAL : Inport
  VINP_NowGearPosnSigOk_flg_InHAL : Inport  Port=2
  VINP_TMTurbineSpdSigOk_flg_InHAL : Inport  Port=3
  Logical Operator{1..4,6,8} (6 alike) : Logic  Operator=NOT  AllPortsSameDT=off  OutDataTypeStr=boolean
  Switch1 : Switch  Criteria=u2 ~= 0
  Switch2 : Switch  Criteria=u2 ~= 0
  Switch3 : Switch  Criteria=u2 ~= 0
  Terminator : Terminator
  Terminator1 : Terminator
  Terminator2 : Terminator
  ovrdflg : Constant  Value=KINP_CANShftPosnSigSigOk_flg_ovrdflg
  ovrdflg1 : Constant  Value=KINP_CANNowGearPosnSigOk_flg_ovrdflg
  ovrdflg2 : Constant  Value=KINP_CANTMTurbSpdSigOk_flg_ovrdflg
  ovrdval : Constant  Value=KINP_CANShftPosnSigOk_flg_ovrdval
  ovrdval1 : Constant  Value=KINP_CANNowGearPosnSigOk_flg_ovrdval
  ovrdval2 : Constant  Value=KINP_CANTMTurbSpdSigOk_flg_ovrdval
  wires:
  VINP_ShifterPosnSigOk_flg_InHAL/1 -> Switch2/1
  Switch2/1 -> Logical Operator6/1
  ovrdflg/1 -> Logical Operator8/1
  ovrdval/1 -> Switch2/3
  Logical Operator8/1 -> Switch2/2
  Logical Operator6/1 -> Terminator/1  "VINP_CANShftPosnSigFault_flg"
  VINP_NowGearPosnSigOk_flg_InHAL/1 -> Switch1/1
  Switch1/1 -> Logical Operator1/1
  ovrdflg1/1 -> Logical Operator2/1
  ovrdval1/1 -> Switch1/3
  Logical Operator2/1 -> Switch1/2
  Logical Operator1/1 -> Terminator1/1  "VINP_CANNowGearPosnSigFault_flg"
  VINP_TMTurbineSpdSigOk_flg_InHAL/1 -> Switch3/1
  Switch3/1 -> Logical Operator3/1
  ovrdflg2/1 -> Logical Operator4/1
  ovrdval2/1 -> Switch3/3
  Logical Operator4/1 -> Switch3/2
  Logical Operator3/1 -> Terminator2/1  "VINP_CANTMTurbSpdSigFault_flg"

== system inp/INP/InputSignals/Diagnosis/VCU_CANSig_Detection ==
  VINP_AckCurBrakDecReqSigOk_flg_InHAL : Inport
  VINP_AckCurPTTqReqSigOk_flg_InHAL : Inport  Port=2
  VINP_AckCurVehDecSigOk_flg_InHAL : Inport  Port=3
  VINP_DriverBrakPedalOnSigOk_flg_InHAL : Inport  Port=4
  VINP_ESCBattVoltSigOk_flg_InHAL : Inport  Port=5
  VINP_HWAccelPedalPosnSigOk_flg_InHAL : Inport  Port=6
  VINP_CurDriverTqReqSigOk_flg_InHAL : Inport  Port=7
  Logical Operator{1..14} (14 alike) : Logic  Operator=NOT  AllPortsSameDT=off  OutDataTypeStr=boolean
  Switch{1..7} (7 alike) : Switch  Criteria=u2 ~= 0
  Terminator{,1..6} (7 alike) : Terminator
  ovrdflg : Constant  Value=KINP_CANAckCurBrakDecReqSigOk_flg_ovrdflg
  ovrdflg1 : Constant  Value=KINP_CANAckCurPTTqReqSigOk_flg_ovrdflg
  ovrdflg2 : Constant  Value=KINP_CANAckCurVehDecSigOk_flg_ovrdflg
  ovrdflg3 : Constant  Value=KINP_CANESCBattVoltSigOk_flg_ovrdflg
  ovrdflg4 : Constant  Value=KINP_CANHWAPPSigOk_flg_ovrdflg
  ovrdflg5 : Constant  Value=KINP_CANCurDriverTqReqSigOk_flg_ovrdflg
  ovrdflg6 : Constant  Value=KINP_CANDriverBrakPedalOnSigOk_flg_ovrdflg
  ovrdval : Constant  Value=KINP_CANAckCurBrakDecReqSigOk_flg_ovrdval
  ovrdval1 : Constant  Value=KINP_CANAckCurPTTqReqSigOk_flg_ovrdval
  ovrdval2 : Constant  Value=KINP_CANAckCurVehDecSigOk_flg_ovrdval
  ovrdval3 : Constant  Value=KINP_CANESCBattVoltSigOk_flg_ovrdval
  ovrdval4 : Constant  Value=KINP_CANHWAPPSigOk_flg_ovrdval
  ovrdval5 : Constant  Value=KINP_CANCurDriverTqReqSigOk_flg_ovrdval
  ovrdval6 : Constant  Value=KINP_CANDriverBrakPedalOnSigOk_flg_ovrdval
  wires:
  VINP_AckCurBrakDecReqSigOk_flg_InHAL/1 -> Switch2/1
  Switch2/1 -> Logical Operator6/1
  ovrdflg/1 -> Logical Operator8/1
  ovrdval/1 -> Switch2/3
  Logical Operator8/1 -> Switch2/2
  Logical Operator6/1 -> Terminator/1  "VINP_CANAckCurBrakDecReqSigFault_flg"
  VINP_AckCurPTTqReqSigOk_flg_InHAL/1 -> Switch1/1
  Switch1/1 -> Logical Operator1/1
  ovrdflg1/1 -> Logical Operator2/1
  ovrdval1/1 -> Switch1/3
  Logical Operator2/1 -> Switch1/2
  Logical Operator1/1 -> Terminator1/1  "VINP_CANAckCurPTTqReqSigFault_flg"
  VINP_AckCurVehDecSigOk_flg_InHAL/1 -> Switch3/1
  Switch3/1 -> Logical Operator3/1
  ovrdflg2/1 -> Logical Operator4/1
  ovrdval2/1 -> Switch3/3
  Logical Operator4/1 -> Switch3/2
  Logical Operator3/1 -> Terminator2/1  "VINP_CANAckCurVehDecSigFault_flg"
  VINP_ESCBattVoltSigOk_flg_InHAL/1 -> Switch4/1
  Switch4/1 -> Logical Operator5/1
  ovrdflg3/1 -> Logical Operator7/1
  ovrdval3/1 -> Switch4/3
  Logical Operator7/1 -> Switch4/2
  Logical Operator5/1 -> Terminator3/1  "VINP_CANESCBattVoltSigFault_flg"
  VINP_HWAccelPedalPosnSigOk_flg_InHAL/1 -> Switch5/1
  Switch5/1 -> Logical Operator9/1
  ovrdflg4/1 -> Logical Operator10/1
  ovrdval4/1 -> Switch5/3
  Logical Operator10/1 -> Switch5/2
  Logical Operator9/1 -> Terminator4/1  "VINP_CANHWAPPSigFault_flg"
  VINP_CurDriverTqReqSigOk_flg_InHAL/1 -> Switch6/1
  Switch6/1 -> Logical Operator12/1
  ovrdflg5/1 -> Logical Operator11/1
  ovrdval5/1 -> Switch6/3
  Logical Operator11/1 -> Switch6/2
  Logical Operator12/1 -> Terminator5/1  "VINP_CANCurDriverTqReqSigFault_flg"
  VINP_DriverBrakPedalOnSigOk_flg_InHAL/1 -> Switch7/1
  Switch7/1 -> Logical Operator13/1
  ovrdflg6/1 -> Logical Operator14/1
  ovrdval6/1 -> Switch7/3
  Logical Operator14/1 -> Switch7/2
  Logical Operator13/1 -> Terminator6/1  "VINP_CANDriverBrkPdlOnSigFault_flg"

== system inp/INP/InputSignals/SignalProcess/AppTimer ==
  Add : Sum
  RESET1 : Constant  Value=5  OutDataTypeStr=single
  Unit Delay : UnitDelay  SampleTime=-1
  VINP_AppTime_ms_InProc : Outport
  wires:
  Add/1 -> Unit Delay/1
  Unit Delay/1 -> VINP_AppTime_ms_InProc/1, Add/1  "VINP_AppTime_ms_InProc"
  RESET1/1 -> Add/2
